simulink model "sin_input1"  (Simulink 7.9)
settings: Solver=ode45  StartTime=0.0  StopTime=50000  RelTol=1e-3
undefined symbols (not defined in the file or in its folder): sfp
libraries: xbsIndex_r4 x2567, xps_library x13, casper_library_misc x4
(part 2 of 28)

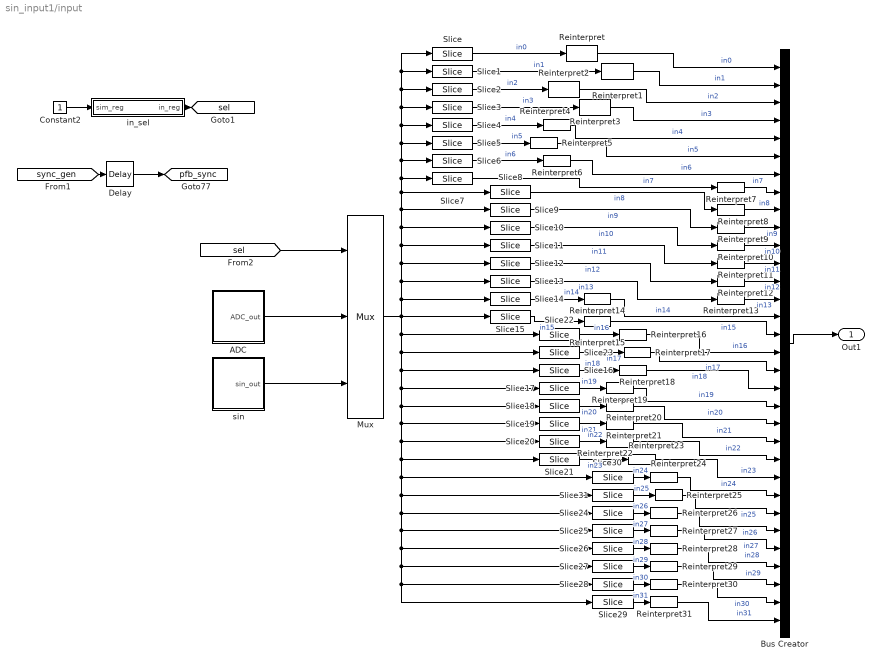
== system sin_input1/input ==
  ADC : Subsystem
  Bus Creator : BusCreator  Inputs=32
  Constant2 : Constant
  Delay : xbsIndex_r4/Delay  rst=off  infoeditControl=Selection of Reset will increase slice count due to use of real FFs and instead of SRLs  en=off  latency=3  dbl_ovrd=off  reg_retiming=off  block_type=delay
  Mux : xbsIndex_r4/Mux  inputs=2  en=off  latency=0  precision=Full  arith_type=Unsigned  n_bits=16  bin_pt=14  quantization=Truncate  overflow=Wrap  dbl_ovrd=off  block_type=mux
  Reinterpret{,1..31} (32 alike) : xbsIndex_r4/Reinterpret  force_arith_type=on  arith_type="Signed  (2's comp)"  force_bin_pt=on  bin_pt=7  block_type=reinterpret
  Slice : xbsIndex_r4/Slice  nbits=8  boolean_output=off  mode=Lower Bit Location + Width  bit1=0  base1=MSB of Input  bit0=248  base0=LSB of Input  dbl_ovrd=off  block_type=slice
  Slice1 : xbsIndex_r4/Slice  nbits=8  boolean_output=off  mode=Lower Bit Location + Width  bit1=0  base1=MSB of Input  bit0=240  base0=LSB of Input  dbl_ovrd=off  block_type=slice
  Slice10 : xbsIndex_r4/Slice  nbits=8  boolean_output=off  mode=Lower Bit Location + Width  bit1=0  base1=MSB of Input  bit0=168  base0=LSB of Input  dbl_ovrd=off  block_type=slice
  Slice11 : xbsIndex_r4/Slice  nbits=8  boolean_output=off  mode=Lower Bit Location + Width  bit1=0  base1=MSB of Input  bit0=160  base0=LSB of Input  dbl_ovrd=off  block_type=slice
  Slice12 : xbsIndex_r4/Slice  nbits=8  boolean_output=off  mode=Lower Bit Location + Width  bit1=0  base1=MSB of Input  bit0=152  base0=LSB of Input  dbl_ovrd=off  block_type=slice
  Slice13 : xbsIndex_r4/Slice  nbits=8  boolean_output=off  mode=Lower Bit Location + Width  bit1=0  base1=MSB of Input  bit0=144  base0=LSB of Input  dbl_ovrd=off  block_type=slice
  Slice14 : xbsIndex_r4/Slice  nbits=8  boolean_output=off  mode=Lower Bit Location + Width  bit1=0  base1=MSB of Input  bit0=136  base0=LSB of Input  dbl_ovrd=off  block_type=slice
  Slice15 : xbsIndex_r4/Slice  nbits=8  boolean_output=off  mode=Lower Bit Location + Width  bit1=0  base1=MSB of Input  bit0=128  base0=LSB of Input  dbl_ovrd=off  block_type=slice
  Slice16 : xbsIndex_r4/Slice  nbits=8  boolean_output=off  mode=Lower Bit Location + Width  bit1=0  base1=MSB of Input  bit0=104  base0=LSB of Input  dbl_ovrd=off  block_type=slice
  Slice17 : xbsIndex_r4/Slice  nbits=8  boolean_output=off  mode=Lower Bit Location + Width  bit1=0  base1=MSB of Input  bit0=96  base0=LSB of Input  dbl_ovrd=off  block_type=slice
  Slice18 : xbsIndex_r4/Slice  nbits=8  boolean_output=off  mode=Lower Bit Location + Width  bit1=0  base1=MSB of Input  bit0=88  base0=LSB of Input  dbl_ovrd=off  block_type=slice
  Slice19 : xbsIndex_r4/Slice  nbits=8  boolean_output=off  mode=Lower Bit Location + Width  bit1=0  base1=MSB of Input  bit0=80  base0=LSB of Input  dbl_ovrd=off  block_type=slice
  Slice2 : xbsIndex_r4/Slice  nbits=8  boolean_output=off  mode=Lower Bit Location + Width  bit1=0  base1=MSB of Input  bit0=232  base0=LSB of Input  dbl_ovrd=off  block_type=slice
  Slice20 : xbsIndex_r4/Slice  nbits=8  boolean_output=off  mode=Lower Bit Location + Width  bit1=0  base1=MSB of Input  bit0=72  base0=LSB of Input  dbl_ovrd=off  block_type=slice
  Slice21 : xbsIndex_r4/Slice  nbits=8  boolean_output=off  mode=Lower Bit Location + Width  bit1=0  base1=MSB of Input  bit0=64  base0=LSB of Input  dbl_ovrd=off  block_type=slice
  Slice22 : xbsIndex_r4/Slice  nbits=8  boolean_output=off  mode=Lower Bit Location + Width  bit1=0  base1=MSB of Input  bit0=120  base0=LSB of Input  dbl_ovrd=off  block_type=slice
  Slice23 : xbsIndex_r4/Slice  nbits=8  boolean_output=off  mode=Lower Bit Location + Width  bit1=0  base1=MSB of Input  bit0=112  base0=LSB of Input  dbl_ovrd=off  block_type=slice
  Slice24 : xbsIndex_r4/Slice  nbits=8  boolean_output=off  mode=Lower Bit Location + Width  bit1=0  base1=MSB of Input  bit0=40  base0=LSB of Input  dbl_ovrd=off  block_type=slice
  Slice25 : xbsIndex_r4/Slice  nbits=8  boolean_output=off  mode=Lower Bit Location + Width  bit1=0  base1=MSB of Input  bit0=32  base0=LSB of Input  dbl_ovrd=off  block_type=slice
  Slice26 : xbsIndex_r4/Slice  nbits=8  boolean_output=off  mode=Lower Bit Location + Width  bit1=0  base1=MSB of Input  bit0=24  base0=LSB of Input  dbl_ovrd=off  block_type=slice
  Slice27 : xbsIndex_r4/Slice  nbits=8  boolean_output=off  mode=Lower Bit Location + Width  bit1=0  base1=MSB of Input  bit0=16  base0=LSB of Input  dbl_ovrd=off  block_type=slice
  Slice28 : xbsIndex_r4/Slice  nbits=8  boolean_output=off  mode=Lower Bit Location + Width  bit1=0  base1=MSB of Input  bit0=8  base0=LSB of Input  dbl_ovrd=off  block_type=slice
  Slice29 : xbsIndex_r4/Slice  nbits=8  boolean_output=off  mode=Lower Bit Location + Width  bit1=0  base1=MSB of Input  bit0=0  base0=LSB of Input  dbl_ovrd=off  block_type=slice
  Slice3 : xbsIndex_r4/Slice  nbits=8  boolean_output=off  mode=Lower Bit Location + Width  bit1=0  base1=MSB of Input  bit0=224  base0=LSB of Input  dbl_ovrd=off  block_type=slice
  Slice30 : xbsIndex_r4/Slice  nbits=8  boolean_output=off  mode=Lower Bit Location + Width  bit1=0  base1=MSB of Input  bit0=56  base0=LSB of Input  dbl_ovrd=off  block_type=slice
  Slice31 : xbsIndex_r4/Slice  nbits=8  boolean_output=off  mode=Lower Bit Location + Width  bit1=0  base1=MSB of Input  bit0=48  base0=LSB of Input  dbl_ovrd=off  block_type=slice
  Slice4 : xbsIndex_r4/Slice  nbits=8  boolean_output=off  mode=Lower Bit Location + Width  bit1=0  base1=MSB of Input  bit0=216  base0=LSB of Input  dbl_ovrd=off  block_type=slice
  Slice5 : xbsIndex_r4/Slice  nbits=8  boolean_output=off  mode=Lower Bit Location + Width  bit1=0  base1=MSB of Input  bit0=208  base0=LSB of Input  dbl_ovrd=off  block_type=slice
  Slice6 : xbsIndex_r4/Slice  nbits=8  boolean_output=off  mode=Lower Bit Location + Width  bit1=0  base1=MSB of Input  bit0=200  base0=LSB of Input  dbl_ovrd=off  block_type=slice
  Slice7 : xbsIndex_r4/Slice  nbits=8  boolean_output=off  mode=Lower Bit Location + Width  bit1=0  base1=MSB of Input  bit0=192  base0=LSB of Input  dbl_ovrd=off  block_type=slice
  Slice8 : xbsIndex_r4/Slice  nbits=8  boolean_output=off  mode=Lower Bit Location + Width  bit1=0  base1=MSB of Input  bit0=184  base0=LSB of Input  dbl_ovrd=off  block_type=slice
  Slice9 : xbsIndex_r4/Slice  nbits=8  boolean_output=off  mode=Lower Bit Location + Width  bit1=0  base1=MSB of Input  bit0=176  base0=LSB of Input  dbl_ovrd=off  block_type=slice
  in_sel : Subsystem  Tag=xps:sw_reg  AncestorBlock=xps_library/software register  mask{numios=1, io_dir=From Processor, io_delay=0, sample_period=1, name1=reg, bitwidth1=1, arith_type1=Boolean, bin_pt1=0}
      mask prompts: numios "Num I/Os"; io_dir "I/O direction"; io_delay "I/O delay"; sample_period "Sample period"; name1 "Name"; bitwidth1 "Bitwidth"; arith_type1 "Type"; bin_pt1 "Binary pt"
      mask init:
      | swreg_init(gcb);
      mask StyleString: edit,popup(To Processor|From Processor),edit,edit,edit,edit,popup(Unsigned|Signed  (2's comp)|Boolean),edit
      mask CallbackString:
      | t = timer('StartDelay', 0.1); t.TimerFcn = {@swreg_cb, gcb}; start(t);|a = get_param(gcb, 'MaskEnables');
      | if strcmp(get_param(gcb, 'io_dir'), 'To Processor'),
      | a(4) = {'off'};
      | else
      | a(4) = {'on'};
      | end
      | set_param(gcb, 'MaskEnables', a);
      | |||||a = get_param(gcb, 'MaskEnables');
      | if strcmp(get_param(gcb, 'arith_type1'), 'Boolean'),
      | set_param(gcb, 'bitwidth1', '1');
      | set_param(gcb, 'bin_pt1', '0');
      | a(6) = {'off'};
      | a(8) = {'off'};
      | else
      | a(6) = {'on'};
      | a(8) = {'on'};
      | end
      | set_param(gcb, 'MaskEnables', a);
      | |
      mask TabNameString: Setup,Setup,Setup,Setup,IO1,IO1,IO1,IO1
  sin : Subsystem
  Out1 : Outport
  tag scope: pfb_sync=global, sel=global
  wires:
  ADC/1(ADC_out) -> Mux/2
  sin/1(sin_out) -> Mux/3
  Mux/1 -> Slice15/1, Slice/1, Slice1/1, Slice2/1, Slice3/1, Slice4/1, Slice5/1, Slice6/1, Slice7/1, Slice8/1, Slice9/1, Slice10/1, Slice11/1, Slice12/1, Slice13/1, Slice14/1, Slice22/1, Slice23/1, Slice16/1, Slice17/1, Slice18/1, Slice19/1, Slice20/1, Slice21/1, Slice30/1, Slice31/1, Slice24/1, Slice25/1, Slice26/1, Slice27/1, Slice29/1, Slice28/1
  Slice/1 -> Reinterpret/1  "in0"
  Slice1/1 -> Reinterpret1/1  "in1"
  Slice2/1 -> Reinterpret2/1  "in2"
  Slice3/1 -> Reinterpret3/1  "in3"
  Slice4/1 -> Reinterpret4/1  "in4"
  Slice5/1 -> Reinterpret5/1  "in5"
  Slice6/1 -> Reinterpret6/1  "in6"
  Slice7/1 -> Reinterpret7/1  "in7"
  Slice8/1 -> Reinterpret8/1  "in8"
  Slice9/1 -> Reinterpret9/1  "in9"
  Slice10/1 -> Reinterpret10/1  "in10"
  Slice11/1 -> Reinterpret11/1  "in11"
  Slice12/1 -> Reinterpret12/1  "in12"
  Slice13/1 -> Reinterpret13/1  "in13"
  Slice14/1 -> Reinterpret14/1  "in14"
  Slice15/1 -> Reinterpret15/1  "in15"
  Slice22/1 -> Reinterpret16/1  "in16"
  Slice17/1 -> Reinterpret19/1  "in19"
  Slice18/1 -> Reinterpret20/1  "in20"
  Slice19/1 -> Reinterpret21/1  "in21"
  Slice20/1 -> Reinterpret22/1  "in22"
  Slice21/1 -> Reinterpret23/1  "in23"
  Slice30/1 -> Reinterpret24/1  "in24"
  Slice31/1 -> Reinterpret25/1  "in25"
  Slice24/1 -> Reinterpret26/1  "in26"
  Slice25/1 -> Reinterpret27/1  "in27"
  Slice26/1 -> Reinterpret28/1  "in28"
  Slice27/1 -> Reinterpret29/1  "in29"
  Slice28/1 -> Reinterpret30/1  "in30"
  Slice29/1 -> Reinterpret31/1  "in31"
  Bus Creator/1 -> Out1/1
  Slice23/1 -> Reinterpret17/1  "in17"
  Slice16/1 -> Reinterpret18/1  "in18"
  Reinterpret/1 -> Bus Creator/1  "in0"
  Reinterpret1/1 -> Bus Creator/2  "in1"
  Reinterpret2/1 -> Bus Creator/3  "in2"
  Reinterpret3/1 -> Bus Creator/4  "in3"
  Reinterpret4/1 -> Bus Creator/5  "in4"
  Reinterpret5/1 -> Bus Creator/6  "in5"
  Reinterpret6/1 -> Bus Creator/7  "in6"
  Reinterpret7/1 -> Bus Creator/8  "in7"
  Reinterpret8/1 -> Bus Creator/9  "in8"
  Reinterpret9/1 -> Bus Creator/10  "in9"
  Reinterpret10/1 -> Bus Creator/11  "in10"
  Reinterpret11/1 -> Bus Creator/12  "in11"
  Reinterpret12/1 -> Bus Creator/13  "in12"
  Reinterpret13/1 -> Bus Creator/14  "in13"
  Reinterpret14/1 -> Bus Creator/15  "in14"
  Reinterpret15/1 -> Bus Creator/16  "in15"
  Reinterpret16/1 -> Bus Creator/17  "in16"
  Reinterpret17/1 -> Bus Creator/18  "in17"
  Reinterpret18/1 -> Bus Creator/19  "in18"
  Reinterpret19/1 -> Bus Creator/20  "in19"
  Reinterpret20/1 -> Bus Creator/21  "in20"
  Reinterpret21/1 -> Bus Creator/22  "in21"
  Reinterpret22/1 -> Bus Creator/23  "in22"
  Reinterpret23/1 -> Bus Creator/24  "in23"
  Reinterpret24/1 -> Bus Creator/25  "in24"
  Reinterpret25/1 -> Bus Creator/26  "in25"
  Reinterpret26/1 -> Bus Creator/27  "in26"
  Reinterpret27/1 -> Bus Creator/28  "in27"
  Reinterpret28/1 -> Bus Creator/29  "in28"
  Reinterpret29/1 -> Bus Creator/30  "in29"
  Reinterpret30/1 -> Bus Creator/31  "in30"
  Reinterpret31/1 -> Bus Creator/32  "in31"
  Delay/1 -> [pfb_sync]
  [sync_gen] -> Delay/1
  Constant2/1 -> in_sel/1(sim_reg)
  in_sel/1(in_reg) -> [sel]
  [sel] -> Mux/1

== system sin_input1/monitor ==
  note: GPIO Select (sync_out)
      | 0: zero
      | 1: sync_gpio_in
      | 2: sync_in
      | 3: adc_clip
      | 4: eq_clip
  note: LED Select
      | 0: Clip (LED1=adc_clip; LED0=eq_clip
      | 1: Sync (LED1=sync_gpio_in; LED0=sync_in)
  note: sel[4] = ledsel
      | sel[2:0] = gpiosel
  BitBasher : xbsIndex_r4/BitBasher  bitexpr=|  display_expr=off  sr_1=1  arith_type1=Unsigned  bin_pt1=0  sr_2=2  arith_type2=Unsigned  bin_pt2=0  sr_3=3  arith_type3=Unsigned  bin_pt3=0  sr_4=4  arith_type4=Unsigned  bin_pt4=0  dbl_ovrd=off  block_type=bitbasher
      | <!DOCTYPE HTML PUBLIC "-//W3C//DTD HTML 4.0//EN" "http://www.w3.org/TR/REC-html40/strict.dtd">
      | <html><head><meta name="qrichtext" content="1" /><style type="text/css">
      | p, li { white-space: pre-wrap; }
      | </style></head><body style=" font-family:'Sans'; font-size:10pt; font-weight:400; font-style:normal;">
      | <p style=" margin-top:12px; margin-bottom:12px; margin-left:0px; margin-right:0px; -qt-block-indent:0; text-indent:0px;"><span style=" font-family:'MS Shell Dlg'; font-size:8pt;">gpiosel={sel[2:0]}<br />ledsel={sel[4]}</span></p></body></html>
  Constant1 : Constant  Value=0
  gpio : Subsystem
  led : Subsystem
  sel : Subsystem  Tag=xps:sw_reg  AncestorBlock=xps_library/software register  mask{numios=1, io_dir=From Processor, io_delay=0, sample_period=1, name1=reg, bitwidth1=32, arith_type1=Unsigned, bin_pt1=0}
      mask prompts: numios "Num I/Os"; io_dir "I/O direction"; io_delay "I/O delay"; sample_period "Sample period"; name1 "Name"; bitwidth1 "Bitwidth"; arith_type1 "Type"; bin_pt1 "Binary pt"
      mask init:
      | swreg_init(gcb);
      mask StyleString: edit,popup(To Processor|From Processor),edit,edit,edit,edit,popup(Unsigned|Signed  (2's comp)|Boolean),edit
      mask CallbackString:
      | t = timer('StartDelay', 0.1); t.TimerFcn = {@swreg_cb, gcb}; start(t);|a = get_param(gcb, 'MaskEnables');
      | if strcmp(get_param(gcb, 'io_dir'), 'To Processor'),
      | a(4) = {'off'};
      | else
      | a(4) = {'on'};
      | end
      | set_param(gcb, 'MaskEnables', a);
      | |||||a = get_param(gcb, 'MaskEnables');
      | if strcmp(get_param(gcb, 'arith_type1'), 'Boolean'),
      | set_param(gcb, 'bitwidth1', '1');
      | set_param(gcb, 'bin_pt1', '0');
      | a(6) = {'off'};
      | a(8) = {'off'};
      | else
      | a(6) = {'on'};
      | a(8) = {'on'};
      | end
      | set_param(gcb, 'MaskEnables', a);
      | |
      mask TabNameString: Setup,Setup,Setup,Setup,IO1,IO1,IO1,IO1
  wires:
  Constant1/1 -> sel/1(sim_reg)
  sel/1(in_reg) -> BitBasher/1
  BitBasher/1 -> [gpiosel]
  BitBasher/2 -> [ledsel]
  [gpiosel] -> gpio/1(sel)
  [ledsel] -> led/1(sel)

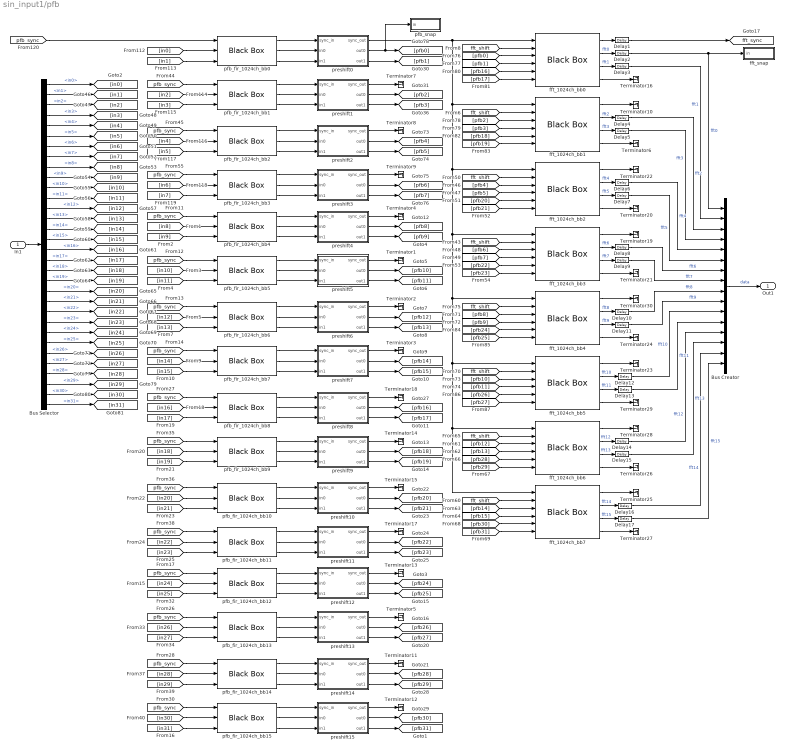
== system sin_input1/pfb ==
  In1 : Inport
  Bus Creator : BusCreator  Inputs=16
  Bus Selector : BusSelector  OutputSignals=in0,in1,in2,in3,in4,in5,in6,in7,in8,in9,in10,in11,in12,in13,in14,in15,in16,in17,in18,in19,in20,in21,in22,in23,in24,in25,in26,in27,in28,in29,in30,in31
  Delay{1..17} (17 alike) : xbsIndex_r4/Delay  rst=off  infoeditControl=Selection of Reset will increase slice count due to use of real FFs and instead of SRLs  en=off  latency=1  dbl_ovrd=off  reg_retiming=off  block_type=delay
  Terminator{1..30} (30 alike) : Terminator
  fft_1024ch_bb{0..7} (8 alike) : xbsIndex_r4/Black Box  init_code=fft_1024ch_core_config  sim_method=Inactive  verbose=off  block_type=blackbox2
  fft_snap : Subsystem
  pfb_fir_1024ch_bb{0..15} (16 alike) : xbsIndex_r4/Black Box  init_code=pfb_fir_1024ch_core_config  sim_method=Inactive  verbose=off  block_type=blackbox2
  pfb_snap : Subsystem
  preshift0 : Subsystem
  preshift1 : Subsystem
  preshift10 : Subsystem
  preshift11 : Subsystem
  preshift12 : Subsystem
  preshift13 : Subsystem
  preshift14 : Subsystem
  preshift15 : Subsystem
  preshift2 : Subsystem
  preshift3 : Subsystem
  preshift4 : Subsystem
  preshift5 : Subsystem
  preshift6 : Subsystem
  preshift7 : Subsystem
  preshift8 : Subsystem
  preshift9 : Subsystem
  Out1 : Outport
  tag scope: fft_sync=global
  wires:
  [in0] -> pfb_fir_1024ch_bb0/2
  [in1] -> pfb_fir_1024ch_bb0/3
  [in2] -> pfb_fir_1024ch_bb1/2
  [in3] -> pfb_fir_1024ch_bb1/3
  [in4] -> pfb_fir_1024ch_bb2/2
  [in5] -> pfb_fir_1024ch_bb2/3
  [in6] -> pfb_fir_1024ch_bb3/2
  [in7] -> pfb_fir_1024ch_bb3/3
  pfb_fir_1024ch_bb1/2 -> preshift1/2(in0)
  pfb_fir_1024ch_bb1/3 -> preshift1/3(in1)
  pfb_fir_1024ch_bb2/2 -> preshift2/2(in0)
  pfb_fir_1024ch_bb2/3 -> preshift2/3(in1)
  pfb_fir_1024ch_bb3/2 -> preshift3/2(in0)
  pfb_fir_1024ch_bb3/3 -> preshift3/3(in1)
  [fft_shift] -> fft_1024ch_bb1/2
  fft_1024ch_bb1/4 -> Terminator6/1
  fft_1024ch_bb0/4 -> Terminator16/1
  Delay1/1 -> [fft_sync]
  fft_1024ch_bb1/1 -> Terminator10/1
  [fft_shift] -> fft_1024ch_bb0/2
  [pfb_sync] -> pfb_fir_1024ch_bb0/1
  [pfb_sync] -> pfb_fir_1024ch_bb1/1
  [pfb_sync] -> pfb_fir_1024ch_bb2/1
  [pfb_sync] -> pfb_fir_1024ch_bb3/1
  pfb_fir_1024ch_bb1/1 -> preshift1/1(sync_in)
  pfb_fir_1024ch_bb2/1 -> preshift2/1(sync_in)
  pfb_fir_1024ch_bb3/1 -> preshift3/1(sync_in)
  pfb_fir_1024ch_bb0/1 -> preshift0/1(sync_in)
  pfb_fir_1024ch_bb0/2 -> preshift0/2(in0)
  pfb_fir_1024ch_bb0/3 -> preshift0/3(in1)
  preshift0/1(sync_out) -> fft_1024ch_bb6/1, fft_1024ch_bb7/1, fft_1024ch_bb5/1, fft_1024ch_bb4/1, fft_1024ch_bb3/1, fft_1024ch_bb2/1, fft_1024ch_bb1/1, fft_1024ch_bb0/1
  preshift0/2(out0) -> [pfb0], pfb_snap/1(in)
  preshift0/3(out1) -> [pfb1]
  preshift1/1(sync_out) -> Terminator7/1
  preshift2/1(sync_out) -> Terminator8/1
  preshift3/1(sync_out) -> Terminator9/1
  preshift1/2(out0) -> [pfb2]
  preshift1/3(out1) -> [pfb3]
  preshift2/2(out0) -> [pfb4]
  preshift2/3(out1) -> [pfb5]
  preshift3/2(out0) -> [pfb6]
  preshift3/3(out1) -> [pfb7]
  [pfb0] -> fft_1024ch_bb0/3
  [pfb1] -> fft_1024ch_bb0/4
  [pfb16] -> fft_1024ch_bb0/5
  [pfb17] -> fft_1024ch_bb0/6
  [pfb2] -> fft_1024ch_bb1/3
  [pfb3] -> fft_1024ch_bb1/4
  [pfb18] -> fft_1024ch_bb1/5
  [pfb19] -> fft_1024ch_bb1/6
  [in8] -> pfb_fir_1024ch_bb4/2
  [in9] -> pfb_fir_1024ch_bb4/3
  [in10] -> pfb_fir_1024ch_bb5/2
  [in11] -> pfb_fir_1024ch_bb5/3
  [in12] -> pfb_fir_1024ch_bb6/2
  [in13] -> pfb_fir_1024ch_bb6/3
  [in14] -> pfb_fir_1024ch_bb7/2
  [in15] -> pfb_fir_1024ch_bb7/3
  pfb_fir_1024ch_bb5/2 -> preshift5/2(in0)
  pfb_fir_1024ch_bb5/3 -> preshift5/3(in1)
  pfb_fir_1024ch_bb6/2 -> preshift6/2(in0)
  pfb_fir_1024ch_bb6/3 -> preshift6/3(in1)
  pfb_fir_1024ch_bb7/2 -> preshift7/2(in0)
  pfb_fir_1024ch_bb7/3 -> preshift7/3(in1)
  [pfb_sync] -> pfb_fir_1024ch_bb4/1
  [pfb_sync] -> pfb_fir_1024ch_bb5/1
  [pfb_sync] -> pfb_fir_1024ch_bb6/1
  [pfb_sync] -> pfb_fir_1024ch_bb7/1
  pfb_fir_1024ch_bb5/1 -> preshift5/1(sync_in)
  pfb_fir_1024ch_bb6/1 -> preshift6/1(sync_in)
  pfb_fir_1024ch_bb7/1 -> preshift7/1(sync_in)
  pfb_fir_1024ch_bb4/1 -> preshift4/1(sync_in)
  pfb_fir_1024ch_bb4/2 -> preshift4/2(in0)
  pfb_fir_1024ch_bb4/3 -> preshift4/3(in1)
  preshift4/1(sync_out) -> Terminator4/1
  preshift4/2(out0) -> [pfb8]
  preshift4/3(out1) -> [pfb9]
  preshift5/1(sync_out) -> Terminator1/1
  preshift6/1(sync_out) -> Terminator2/1
  preshift7/1(sync_out) -> Terminator3/1
  preshift5/2(out0) -> [pfb10]
  preshift5/3(out1) -> [pfb11]
  preshift6/2(out0) -> [pfb12]
  preshift6/3(out1) -> [pfb13]
  preshift7/2(out0) -> [pfb14]
  preshift7/3(out1) -> [pfb15]
  [in16] -> pfb_fir_1024ch_bb8/2
  [in17] -> pfb_fir_1024ch_bb8/3
  [in18] -> pfb_fir_1024ch_bb9/2
  [in19] -> pfb_fir_1024ch_bb9/3
  [in20] -> pfb_fir_1024ch_bb10/2
  [in21] -> pfb_fir_1024ch_bb10/3
  [in22] -> pfb_fir_1024ch_bb11/2
  [in23] -> pfb_fir_1024ch_bb11/3
  pfb_fir_1024ch_bb9/2 -> preshift9/2(in0)
  pfb_fir_1024ch_bb9/3 -> preshift9/3(in1)
  pfb_fir_1024ch_bb10/2 -> preshift10/2(in0)
  pfb_fir_1024ch_bb10/3 -> preshift10/3(in1)
  pfb_fir_1024ch_bb11/2 -> preshift11/2(in0)
  pfb_fir_1024ch_bb11/3 -> preshift11/3(in1)
  [pfb_sync] -> pfb_fir_1024ch_bb8/1
  [pfb_sync] -> pfb_fir_1024ch_bb9/1
  [pfb_sync] -> pfb_fir_1024ch_bb10/1
  [pfb_sync] -> pfb_fir_1024ch_bb11/1
  pfb_fir_1024ch_bb9/1 -> preshift9/1(sync_in)
  pfb_fir_1024ch_bb10/1 -> preshift10/1(sync_in)
  pfb_fir_1024ch_bb11/1 -> preshift11/1(sync_in)
  pfb_fir_1024ch_bb8/1 -> preshift8/1(sync_in)
  pfb_fir_1024ch_bb8/2 -> preshift8/2(in0)
  pfb_fir_1024ch_bb8/3 -> preshift8/3(in1)
  preshift8/1(sync_out) -> Terminator18/1
  preshift8/2(out0) -> [pfb16]
  preshift8/3(out1) -> [pfb17]
  preshift9/1(sync_out) -> Terminator14/1
  preshift10/1(sync_out) -> Terminator15/1
  preshift11/1(sync_out) -> Terminator17/1
  preshift9/2(out0) -> [pfb18]
  preshift9/3(out1) -> [pfb19]
  preshift10/2(out0) -> [pfb20]
  preshift10/3(out1) -> [pfb21]
  preshift11/2(out0) -> [pfb22]
  preshift11/3(out1) -> [pfb23]
  [in24] -> pfb_fir_1024ch_bb12/2
  [in25] -> pfb_fir_1024ch_bb12/3
  [in26] -> pfb_fir_1024ch_bb13/2
  [in27] -> pfb_fir_1024ch_bb13/3
  [in28] -> pfb_fir_1024ch_bb14/2
  [in29] -> pfb_fir_1024ch_bb14/3
  [in30] -> pfb_fir_1024ch_bb15/2
  [in31] -> pfb_fir_1024ch_bb15/3
  pfb_fir_1024ch_bb13/2 -> preshift13/2(in0)
  pfb_fir_1024ch_bb13/3 -> preshift13/3(in1)
  pfb_fir_1024ch_bb14/2 -> preshift14/2(in0)
  pfb_fir_1024ch_bb14/3 -> preshift14/3(in1)
  pfb_fir_1024ch_bb15/2 -> preshift15/2(in0)
  pfb_fir_1024ch_bb15/3 -> preshift15/3(in1)
  [pfb_sync] -> pfb_fir_1024ch_bb12/1
  [pfb_sync] -> pfb_fir_1024ch_bb13/1
  [pfb_sync] -> pfb_fir_1024ch_bb14/1
  [pfb_sync] -> pfb_fir_1024ch_bb15/1
  pfb_fir_1024ch_bb13/1 -> preshift13/1(sync_in)
  pfb_fir_1024ch_bb14/1 -> preshift14/1(sync_in)
  pfb_fir_1024ch_bb15/1 -> preshift15/1(sync_in)
  pfb_fir_1024ch_bb12/1 -> preshift12/1(sync_in)
  pfb_fir_1024ch_bb12/2 -> preshift12/2(in0)
  pfb_fir_1024ch_bb12/3 -> preshift12/3(in1)
  preshift12/1(sync_out) -> Terminator13/1
  preshift12/2(out0) -> [pfb24]
  preshift12/3(out1) -> [pfb25]
  preshift13/1(sync_out) -> Terminator5/1
  preshift14/1(sync_out) -> Terminator11/1
  preshift15/1(sync_out) -> Terminator12/1
  preshift13/2(out0) -> [pfb26]
  preshift13/3(out1) -> [pfb27]
  preshift14/2(out0) -> [pfb28]
  preshift14/3(out1) -> [pfb29]
  preshift15/2(out0) -> [pfb30]
  preshift15/3(out1) -> [pfb31]
  [fft_shift] -> fft_1024ch_bb3/2
  fft_1024ch_bb3/4 -> Terminator21/1
  fft_1024ch_bb2/4 -> Terminator20/1
  fft_1024ch_bb2/1 -> Terminator22/1
  fft_1024ch_bb3/1 -> Terminator19/1
  [fft_shift] -> fft_1024ch_bb2/2
  [pfb4] -> fft_1024ch_bb2/3
  [pfb5] -> fft_1024ch_bb2/4
  [pfb20] -> fft_1024ch_bb2/5
  [pfb21] -> fft_1024ch_bb2/6
  [pfb6] -> fft_1024ch_bb3/3
  [pfb7] -> fft_1024ch_bb3/4
  [pfb22] -> fft_1024ch_bb3/5
  [pfb23] -> fft_1024ch_bb3/6
  [fft_shift] -> fft_1024ch_bb5/2
  fft_1024ch_bb5/4 -> Terminator29/1
  fft_1024ch_bb4/4 -> Terminator24/1
  fft_1024ch_bb4/1 -> Terminator30/1
  fft_1024ch_bb5/1 -> Terminator23/1
  [fft_shift] -> fft_1024ch_bb4/2
  [pfb8] -> fft_1024ch_bb4/3
  [pfb9] -> fft_1024ch_bb4/4
  [pfb24] -> fft_1024ch_bb4/5
  [pfb25] -> fft_1024ch_bb4/6
  [pfb10] -> fft_1024ch_bb5/3
  [pfb11] -> fft_1024ch_bb5/4
  [pfb26] -> fft_1024ch_bb5/5
  [pfb27] -> fft_1024ch_bb5/6
  [fft_shift] -> fft_1024ch_bb7/2
  fft_1024ch_bb7/4 -> Terminator27/1
  fft_1024ch_bb6/4 -> Terminator26/1
  fft_1024ch_bb6/1 -> Terminator28/1
  fft_1024ch_bb7/1 -> Terminator25/1
  [fft_shift] -> fft_1024ch_bb6/2
  [pfb12] -> fft_1024ch_bb6/3
  [pfb13] -> fft_1024ch_bb6/4
  [pfb28] -> fft_1024ch_bb6/5
  [pfb29] -> fft_1024ch_bb6/6
  [pfb14] -> fft_1024ch_bb7/3
  [pfb15] -> fft_1024ch_bb7/4
  [pfb30] -> fft_1024ch_bb7/5
  [pfb31] -> fft_1024ch_bb7/6
  In1/1 -> Bus Selector/1
  Bus Selector/1 -> [in0]  "<in0>"
  Bus Selector/2 -> [in1]  "<in1>"
  Bus Selector/3 -> [in2]  "<in2>"
  Bus Selector/4 -> [in3]  "<in3>"
  Delay2/1 -> Bus Creator/1, fft_snap/1(in)  "fft0"
  Delay3/1 -> Bus Creator/2  "fft1"
  Delay4/1 -> Bus Creator/3  "fft2"
  Delay5/1 -> Bus Creator/4  "fft3"
  Delay6/1 -> Bus Creator/5  "fft4"
  Delay7/1 -> Bus Creator/6  "fft5"
  Delay8/1 -> Bus Creator/7  "fft6"
  Delay9/1 -> Bus Creator/8  "fft7"
  Delay10/1 -> Bus Creator/9  "fft8"
  Delay11/1 -> Bus Creator/10  "fft9"
  Delay12/1 -> Bus Creator/11  "fft10"
  Delay13/1 -> Bus Creator/12  "fft11"
  Delay14/1 -> Bus Creator/13  "fft12"
  Delay15/1 -> Bus Creator/14  "fft13"
  Delay16/1 -> Bus Creator/15  "fft14"
  Delay17/1 -> Bus Creator/16  "fft15"
  Bus Creator/1 -> Out1/1  "data"
  fft_1024ch_bb7/2 -> Delay16/1  "fft14"
  fft_1024ch_bb7/3 -> Delay17/1  "fft15"
  fft_1024ch_bb6/2 -> Delay14/1  "fft12"
  fft_1024ch_bb6/3 -> Delay15/1  "fft13"
  fft_1024ch_bb5/2 -> Delay12/1  "fft10"
  fft_1024ch_bb5/3 -> Delay13/1  "fft11"
  fft_1024ch_bb0/2 -> Delay2/1  "fft0"
  fft_1024ch_bb0/1 -> Delay1/1
  fft_1024ch_bb0/3 -> Delay3/1  "fft1"
  fft_1024ch_bb1/2 -> Delay4/1  "fft2"
  fft_1024ch_bb1/3 -> Delay5/1  "fft3"
  fft_1024ch_bb2/2 -> Delay6/1  "fft4"
  fft_1024ch_bb2/3 -> Delay7/1  "fft5"
  fft_1024ch_bb3/2 -> Delay8/1  "fft6"
  fft_1024ch_bb3/3 -> Delay9/1  "fft7"
  fft_1024ch_bb4/2 -> Delay10/1  "fft8"
  fft_1024ch_bb4/3 -> Delay11/1  "fft9"
  Bus Selector/5 -> [in4]  "<in4>"
  Bus Selector/6 -> [in5]  "<in5>"
  Bus Selector/7 -> [in6]  "<in6>"
  Bus Selector/8 -> [in7]  "<in7>"
  Bus Selector/9 -> [in8]  "<in8>"
  Bus Selector/10 -> [in9]  "<in9>"
  Bus Selector/11 -> [in10]  "<in10>"
  Bus Selector/12 -> [in11]  "<in11>"
  Bus Selector/13 -> [in12]  "<in12>"
  Bus Selector/14 -> [in13]  "<in13>"
  Bus Selector/15 -> [in14]  "<in14>"
  Bus Selector/16 -> [in15]  "<in15>"
  Bus Selector/17 -> [in16]  "<in16>"
  Bus Selector/18 -> [in17]  "<in17>"
  Bus Selector/19 -> [in18]  "<in18>"
  Bus Selector/20 -> [in19]  "<in19>"
  Bus Selector/21 -> [in20]  "<in20>"
  Bus Selector/22 -> [in21]  "<in21>"
  Bus Selector/23 -> [in22]  "<in22>"
  Bus Selector/24 -> [in23]  "<in23>"
  Bus Selector/25 -> [in24]  "<in24>"
  Bus Selector/26 -> [in25]  "<in25>"
  Bus Selector/27 -> [in26]  "<in26>"
  Bus Selector/28 -> [in27]  "<in27>"
  Bus Selector/29 -> [in28]  "<in28>"
  Bus Selector/30 -> [in29]  "<in29>"
  Bus Selector/31 -> [in30]  "<in30>"
  Bus Selector/32 -> [in31]  "<in31>"

== system sin_input1/rcs ==
  note: 0 -> revision info
      | 1 -> timestamp
  note: Last modification
      | in seconds since
      | 01-01-1970
  note: 0 -> git
      | 1 -> svn
  note: 0 -> cleanly in revision control
  note: git -> first 28 bits of commit hash
      | svn -> latest revision number
  note: 0 -> revision info
      | 1 -> timestamp
  note: Compile time
      | in seconds since
      | 01-01-1970
  note: 0 -> git
      | 1 -> svn
  note: 0 -> cleanly in revision control
  note: git -> first 28 bits of commit hash
      | svn -> latest revision number
  Concat : xbsIndex_r4/Concat  num_inputs=2  dbl_ovrd=off  block_type=concat
  Concat1 : xbsIndex_r4/Concat  num_inputs=2  dbl_ovrd=off  block_type=concat
  Mux : xbsIndex_r4/Mux  inputs=2  en=off  latency=0  precision=Full  arith_type=Unsigned  n_bits=16  bin_pt=14  quantization=Truncate  overflow=Wrap  dbl_ovrd=off  block_type=mux
      settings: block_version=11.4
  Mux1 : xbsIndex_r4/Mux  inputs=2  en=off  latency=0  precision=Full  arith_type=Unsigned  n_bits=16  bin_pt=14  quantization=Truncate  overflow=Wrap  dbl_ovrd=off  block_type=mux
      settings: block_version=11.4
  Terminator : Terminator
  Terminator1 : Terminator
  Terminator2 : Terminator
  app : Subsystem  Tag=xps:sw_reg  mask{io_dir=To Processor, arith_type=Unsigned, bitwidth=32, bin_pt=0, sample_period=1, latency=0}
      mask prompts: io_dir "I/O direction"; arith_type "Data Type"; bitwidth "Data bitwidth"; bin_pt "Data binary point"; sample_period "Sample period"
      mask init:
      | gw_name = clear_name(gcb);
      | config.source = str2func('software_register_init');
      | config.toplevel = gcb;
      | xBlock( config, {io_dir, arith_type, bitwidth, bin_pt, ... 
      |     sample_period, gw_name, latency});
      mask StyleString: popup(From Processor|To Processor),popup(Unsigned|Signed  (2's comp)),edit,edit,edit,edit
  app_dirty : xbsIndex_r4/Constant  const=1  gui_display_data_type=Fixed-point  arith_type=Unsigned  n_bits=1  bin_pt=0  preci_type=Single  exp_width=8  frac_width=24  explicit_period=on  period=1  equ=P=C  opselect=C  inp2=PCIN>>17  opr=+  inp1=P  carry=CIN  dbl_ovrd=off  block_type=constant
      settings: block_version=11.4
  app_info : xbsIndex_r4/Concat  num_inputs=4  dbl_ovrd=off  block_type=concat
  app_info1 : xbsIndex_r4/Concat  num_inputs=4  dbl_ovrd=off  block_type=concat
  app_rcs_type : xbsIndex_r4/Constant  const=0  gui_display_data_type=Fixed-point  arith_type=Unsigned  n_bits=1  bin_pt=0  preci_type=Single  exp_width=8  frac_width=24  explicit_period=on  period=1  equ=P=C  opselect=C  inp2=PCIN>>17  opr=+  inp1=P  carry=CIN  dbl_ovrd=off  block_type=constant
      settings: block_version=11.4
  app_reserved : xbsIndex_r4/Constant  const=0  gui_display_data_type=Fixed-point  arith_type=Unsigned  n_bits=1  bin_pt=0  preci_type=Single  exp_width=8  frac_width=24  explicit_period=on  period=1  equ=P=C  opselect=C  inp2=PCIN>>17  opr=+  inp1=P  carry=CIN  dbl_ovrd=off  block_type=constant
      settings: block_version=11.4
  app_revision : xbsIndex_r4/Constant  const=0  gui_display_data_type=Fixed-point  arith_type=Unsigned  n_bits=28  bin_pt=0  preci_type=Single  exp_width=8  frac_width=24  explicit_period=on  period=1  equ=P=C  opselect=C  inp2=PCIN>>17  opr=+  inp1=P  carry=CIN  dbl_ovrd=off  block_type=constant
      settings: block_version=11.4
  app_timestamp : xbsIndex_r4/Constant  const=1445516336  gui_display_data_type=Fixed-point  arith_type=Unsigned  n_bits=31  bin_pt=0  preci_type=Single  exp_width=8  frac_width=24  explicit_period=on  period=1  equ=P=C  opselect=C  inp2=PCIN>>17  opr=+  inp1=P  carry=CIN  dbl_ovrd=off  block_type=constant
      settings: block_version=11.4
  app_type : xbsIndex_r4/Constant  const=1  gui_display_data_type=Fixed-point  arith_type=Unsigned  n_bits=1  bin_pt=0  preci_type=Single  exp_width=8  frac_width=24  explicit_period=on  period=1  equ=P=C  opselect=C  inp2=PCIN>>17  opr=+  inp1=P  carry=CIN  dbl_ovrd=off  block_type=constant
      settings: block_version=11.4
  lib : Subsystem  Tag=xps:sw_reg  mask{io_dir=To Processor, arith_type=Unsigned, bitwidth=32, bin_pt=0, sample_period=1, latency=0}
      mask prompts: io_dir "I/O direction"; arith_type "Data Type"; bitwidth "Data bitwidth"; bin_pt "Data binary point"; sample_period "Sample period"
      mask init:
      | gw_name = clear_name(gcb);
      | config.source = str2func('software_register_init');
      | config.toplevel = gcb;
      | xBlock( config, {io_dir, arith_type, bitwidth, bin_pt, ... 
      |     sample_period, gw_name, latency});
      mask StyleString: popup(From Processor|To Processor),popup(Unsigned|Signed  (2's comp)),edit,edit,edit,edit
  lib_dirty : xbsIndex_r4/Constant  const=1  gui_display_data_type=Fixed-point  arith_type=Unsigned  n_bits=1  bin_pt=0  preci_type=Single  exp_width=8  frac_width=24  explicit_period=on  period=1  equ=P=C  opselect=C  inp2=PCIN>>17  opr=+  inp1=P  carry=CIN  dbl_ovrd=off  block_type=constant
      settings: block_version=11.4
  lib_rcs_type : xbsIndex_r4/Constant  const=0  gui_display_data_type=Fixed-point  arith_type=Unsigned  n_bits=1  bin_pt=0  preci_type=Single  exp_width=8  frac_width=24  explicit_period=on  period=1  equ=P=C  opselect=C  inp2=PCIN>>17  opr=+  inp1=P  carry=CIN  dbl_ovrd=off  block_type=constant
      settings: block_version=11.4
  lib_reserved : xbsIndex_r4/Constant  const=0  gui_display_data_type=Fixed-point  arith_type=Unsigned  n_bits=1  bin_pt=0  preci_type=Single  exp_width=8  frac_width=24  explicit_period=on  period=1  equ=P=C  opselect=C  inp2=PCIN>>17  opr=+  inp1=P  carry=CIN  dbl_ovrd=off  block_type=constant
      settings: block_version=11.4
  lib_revision : xbsIndex_r4/Constant  const=hex2dec('2f40dad')  gui_display_data_type=Fixed-point  arith_type=Unsigned  n_bits=28  bin_pt=0  preci_type=Single  exp_width=8  frac_width=24  explicit_period=on  period=1  equ=P=C  opselect=C  inp2=PCIN>>17  opr=+  inp1=P  carry=CIN  dbl_ovrd=off  block_type=constant
      settings: block_version=11.4
  lib_timestamp : xbsIndex_r4/Constant  const=1445545304  gui_display_data_type=Fixed-point  arith_type=Unsigned  n_bits=31  bin_pt=0  preci_type=Single  exp_width=8  frac_width=24  explicit_period=on  period=1  equ=P=C  opselect=C  inp2=PCIN>>17  opr=+  inp1=P  carry=CIN  dbl_ovrd=off  block_type=constant
      settings: block_version=11.4
  lib_type : xbsIndex_r4/Constant  const=0  gui_display_data_type=Fixed-point  arith_type=Unsigned  n_bits=1  bin_pt=0  preci_type=Single  exp_width=8  frac_width=24  explicit_period=on  period=1  equ=P=C  opselect=C  inp2=PCIN>>17  opr=+  inp1=P  carry=CIN  dbl_ovrd=off  block_type=constant
      settings: block_version=11.4
  user : Subsystem  Tag=xps:sw_reg  mask{io_dir=To Processor, arith_type=Unsigned, bitwidth=32, bin_pt=0, sample_period=1, latency=0}
      mask prompts: io_dir "I/O direction"; arith_type "Data Type"; bitwidth "Data bitwidth"; bin_pt "Data binary point"; sample_period "Sample period"
      mask init:
      | gw_name = clear_name(gcb);
      | config.source = str2func('software_register_init');
      | config.toplevel = gcb;
      | xBlock( config, {io_dir, arith_type, bitwidth, bin_pt, ... 
      |     sample_period, gw_name, latency});
      mask StyleString: popup(From Processor|To Processor),popup(Unsigned|Signed  (2's comp)),edit,edit,edit,edit
  user_val : xbsIndex_r4/Constant  const=user_val  gui_display_data_type=Fixed-point  arith_type=Unsigned  n_bits=32  bin_pt=0  preci_type=Single  exp_width=8  frac_width=24  explicit_period=off  period=1  equ=P=C  opselect=C  inp2=PCIN>>17  opr=+  inp1=P  carry=CIN  dbl_ovrd=off  block_type=constant
      settings: block_version=11.4
  wires:
  app/1(sim_reg_out) -> Terminator/1
  user/1(sim_reg_out) -> Terminator2/1
  user_val/1 -> user/1(reg_out)
  app_rcs_type/1 -> app_info/1
  app_reserved/1 -> app_info/2
  app_dirty/1 -> app_info/3
  app_revision/1 -> app_info/4
  app_type/1 -> Concat/1, Mux/1
  app_info/1 -> Mux/2
  app_timestamp/1 -> Mux/3
  Mux/1 -> Concat/2
  Concat/1 -> app/1(reg_out)
  lib/1(sim_reg_out) -> Terminator1/1
  lib_rcs_type/1 -> app_info1/1
  lib_reserved/1 -> app_info1/2
  lib_dirty/1 -> app_info1/3
  lib_revision/1 -> app_info1/4
  lib_type/1 -> Concat1/1, Mux1/1
  app_info1/1 -> Mux1/2
  lib_timestamp/1 -> Mux1/3
  Mux1/1 -> Concat1/2
  Concat1/1 -> lib/1(reg_out)

== system sin_input1/seed ==
  0 : Subsystem  Tag=xps:sw_reg  AncestorBlock=xps_library/software register  mask{numios=1, io_dir=From Processor, io_delay=0, sample_period=1, name1=reg, bitwidth1=32, arith_type1=Unsigned, bin_pt1=0}
      mask prompts: numios "Num I/Os"; io_dir "I/O direction"; io_delay "I/O delay"; sample_period "Sample period"; name1 "Name"; bitwidth1 "Bitwidth"; arith_type1 "Type"; bin_pt1 "Binary pt"
      mask init:
      | swreg_init(gcb);
      mask StyleString: edit,popup(To Processor|From Processor),edit,edit,edit,edit,popup(Unsigned|Signed  (2's comp)|Boolean),edit
      mask CallbackString:
      | t = timer('StartDelay', 0.1); t.TimerFcn = {@swreg_cb, gcb}; start(t);|a = get_param(gcb, 'MaskEnables');
      | if strcmp(get_param(gcb, 'io_dir'), 'To Processor'),
      | a(4) = {'off'};
      | else
      | a(4) = {'on'};
      | end
      | set_param(gcb, 'MaskEnables', a);
      | |||||a = get_param(gcb, 'MaskEnables');
      | if strcmp(get_param(gcb, 'arith_type1'), 'Boolean'),
      | set_param(gcb, 'bitwidth1', '1');
      | set_param(gcb, 'bin_pt1', '0');
      | a(6) = {'off'};
      | a(8) = {'off'};
      | else
      | a(6) = {'on'};
      | a(8) = {'on'};
      | end
      | set_param(gcb, 'MaskEnables', a);
      | |
      mask TabNameString: Setup,Setup,Setup,Setup,IO1,IO1,IO1,IO1
  1 : Subsystem  Tag=xps:sw_reg  AncestorBlock=xps_library/software register  mask{numios=1, io_dir=From Processor, io_delay=0, sample_period=1, name1=reg, bitwidth1=32, arith_type1=Unsigned, bin_pt1=0}
      mask prompts: numios "Num I/Os"; io_dir "I/O direction"; io_delay "I/O delay"; sample_period "Sample period"; name1 "Name"; bitwidth1 "Bitwidth"; arith_type1 "Type"; bin_pt1 "Binary pt"
      mask init:
      | swreg_init(gcb);
      mask StyleString: edit,popup(To Processor|From Processor),edit,edit,edit,edit,popup(Unsigned|Signed  (2's comp)|Boolean),edit
      mask CallbackString:
      | t = timer('StartDelay', 0.1); t.TimerFcn = {@swreg_cb, gcb}; start(t);|a = get_param(gcb, 'MaskEnables');
      | if strcmp(get_param(gcb, 'io_dir'), 'To Processor'),
      | a(4) = {'off'};
      | else
      | a(4) = {'on'};
      | end
      | set_param(gcb, 'MaskEnables', a);
      | |||||a = get_param(gcb, 'MaskEnables');
      | if strcmp(get_param(gcb, 'arith_type1'), 'Boolean'),
      | set_param(gcb, 'bitwidth1', '1');
      | set_param(gcb, 'bin_pt1', '0');
      | a(6) = {'off'};
      | a(8) = {'off'};
      | else
      | a(6) = {'on'};
      | a(8) = {'on'};
      | end
      | set_param(gcb, 'MaskEnables', a);
      | |
      mask TabNameString: Setup,Setup,Setup,Setup,IO1,IO1,IO1,IO1
  2 : Subsystem  Tag=xps:sw_reg  AncestorBlock=xps_library/software register  mask{numios=1, io_dir=From Processor, io_delay=0, sample_period=1, name1=reg, bitwidth1=32, arith_type1=Unsigned, bin_pt1=0}
      mask prompts: numios "Num I/Os"; io_dir "I/O direction"; io_delay "I/O delay"; sample_period "Sample period"; name1 "Name"; bitwidth1 "Bitwidth"; arith_type1 "Type"; bin_pt1 "Binary pt"
      mask init:
      | swreg_init(gcb);
      mask StyleString: edit,popup(To Processor|From Processor),edit,edit,edit,edit,popup(Unsigned|Signed  (2's comp)|Boolean),edit
      mask CallbackString:
      | t = timer('StartDelay', 0.1); t.TimerFcn = {@swreg_cb, gcb}; start(t);|a = get_param(gcb, 'MaskEnables');
      | if strcmp(get_param(gcb, 'io_dir'), 'To Processor'),
      | a(4) = {'off'};
      | else
      | a(4) = {'on'};
      | end
      | set_param(gcb, 'MaskEnables', a);
      | |||||a = get_param(gcb, 'MaskEnables');
      | if strcmp(get_param(gcb, 'arith_type1'), 'Boolean'),
      | set_param(gcb, 'bitwidth1', '1');
      | set_param(gcb, 'bin_pt1', '0');
      | a(6) = {'off'};
      | a(8) = {'off'};
      | else
      | a(6) = {'on'};
      | a(8) = {'on'};
      | end
      | set_param(gcb, 'MaskEnables', a);
      | |
      mask TabNameString: Setup,Setup,Setup,Setup,IO1,IO1,IO1,IO1
  3 : Subsystem  Tag=xps:sw_reg  AncestorBlock=xps_library/software register  mask{numios=1, io_dir=From Processor, io_delay=0, sample_period=1, name1=reg, bitwidth1=32, arith_type1=Unsigned, bin_pt1=0}
      mask prompts: numios "Num I/Os"; io_dir "I/O direction"; io_delay "I/O delay"; sample_period "Sample period"; name1 "Name"; bitwidth1 "Bitwidth"; arith_type1 "Type"; bin_pt1 "Binary pt"
      mask init:
      | swreg_init(gcb);
      mask StyleString: edit,popup(To Processor|From Processor),edit,edit,edit,edit,popup(Unsigned|Signed  (2's comp)|Boolean),edit
      mask CallbackString:
      | t = timer('StartDelay', 0.1); t.TimerFcn = {@swreg_cb, gcb}; start(t);|a = get_param(gcb, 'MaskEnables');
      | if strcmp(get_param(gcb, 'io_dir'), 'To Processor'),
      | a(4) = {'off'};
      | else
      | a(4) = {'on'};
      | end
      | set_param(gcb, 'MaskEnables', a);
      | |||||a = get_param(gcb, 'MaskEnables');
      | if strcmp(get_param(gcb, 'arith_type1'), 'Boolean'),
      | set_param(gcb, 'bitwidth1', '1');
      | set_param(gcb, 'bin_pt1', '0');
      | a(6) = {'off'};
      | a(8) = {'off'};
      | else
      | a(6) = {'on'};
      | a(8) = {'on'};
      | end
      | set_param(gcb, 'MaskEnables', a);
      | |
      mask TabNameString: Setup,Setup,Setup,Setup,IO1,IO1,IO1,IO1
  BitBasher : xbsIndex_r4/BitBasher  bitexpr=|  display_expr=off  sr_1=1  arith_type1=Unsigned  bin_pt1=0  sr_2=2  arith_type2=Unsigned  bin_pt2=0  sr_3=3  arith_type3=Unsigned  bin_pt3=0  sr_4=4  arith_type4=Unsigned  bin_pt4=0  dbl_ovrd=off  block_type=bitbasher
      | <!DOCTYPE HTML PUBLIC "-//W3C//DTD HTML 4.0//EN" "http://www.w3.org/TR/REC-html40/strict.dtd">
      | <html><head><meta name="qrichtext" content="1" /><style type="text/css">
      | p, li { white-space: pre-wrap; }
      | </style></head><body style=" font-family:'Sans'; font-size:10pt; font-weight:400; font-style:normal;">
      | <p style=" margin-top:12px; margin-bottom:12px; margin-left:0px; margin-right:0px; -qt-block-indent:0; text-indent:0px;"><span style=" font-family:'MS Shell Dlg'; font-size:8pt;">s0={8'h5c, s32[7:0], s32[0:7], 8'ha3}<br />s1={8'h5c, s32[15:8], s32[8:15], 8'ha3}<br />s2={8'h5c, s32[23:16], s32[16:23], 8'ha3}<br />s3={8'h5c, s32[31:24], s32[24:31], 8'ha3}</span></p></body></html>
  BitBasher1 : xbsIndex_r4/BitBasher  bitexpr=|  display_expr=off  sr_1=1  arith_type1=Unsigned  bin_pt1=0  sr_2=2  arith_type2=Unsigned  bin_pt2=0  sr_3=3  arith_type3=Unsigned  bin_pt3=0  sr_4=4  arith_type4=Unsigned  bin_pt4=0  dbl_ovrd=off  block_type=bitbasher
      | <!DOCTYPE HTML PUBLIC "-//W3C//DTD HTML 4.0//EN" "http://www.w3.org/TR/REC-html40/strict.dtd">
      | <html><head><meta name="qrichtext" content="1" /><style type="text/css">
      | p, li { white-space: pre-wrap; }
      | </style></head><body style=" font-family:'Sans'; font-size:10pt; font-weight:400; font-style:normal;">
      | <p style=" margin-top:12px; margin-bottom:12px; margin-left:0px; margin-right:0px; -qt-block-indent:0; text-indent:0px;"><span style=" font-family:'MS Shell Dlg'; font-size:8pt;">s0={8'h5c, s32[7:0], s32[0:7], 8'ha3}<br />s1={8'h5c, s32[15:8], s32[8:15], 8'ha3}<br />s2={8'h5c, s32[23:16], s32[16:23], 8'ha3}<br />s3={8'h5c, s32[31:24], s32[24:31], 8'ha3}</span></p></body></html>
  BitBasher2 : xbsIndex_r4/BitBasher  bitexpr=|  display_expr=off  sr_1=1  arith_type1=Unsigned  bin_pt1=0  sr_2=2  arith_type2=Unsigned  bin_pt2=0  sr_3=3  arith_type3=Unsigned  bin_pt3=0  sr_4=4  arith_type4=Unsigned  bin_pt4=0  dbl_ovrd=off  block_type=bitbasher
      | <!DOCTYPE HTML PUBLIC "-//W3C//DTD HTML 4.0//EN" "http://www.w3.org/TR/REC-html40/strict.dtd">
      | <html><head><meta name="qrichtext" content="1" /><style type="text/css">
      | p, li { white-space: pre-wrap; }
      | </style></head><body style=" font-family:'Sans'; font-size:10pt; font-weight:400; font-style:normal;">
      | <p style=" margin-top:12px; margin-bottom:12px; margin-left:0px; margin-right:0px; -qt-block-indent:0; text-indent:0px;"><span style=" font-family:'MS Shell Dlg'; font-size:8pt;">s0={8'h5c, s32[7:0], s32[0:7], 8'ha3}<br />s1={8'h5c, s32[15:8], s32[8:15], 8'ha3}<br />s2={8'h5c, s32[23:16], s32[16:23], 8'ha3}<br />s3={8'h5c, s32[31:24], s32[24:31], 8'ha3}</span></p></body></html>
  BitBasher3 : xbsIndex_r4/BitBasher  bitexpr=|  display_expr=off  sr_1=1  arith_type1=Unsigned  bin_pt1=0  sr_2=2  arith_type2=Unsigned  bin_pt2=0  sr_3=3  arith_type3=Unsigned  bin_pt3=0  sr_4=4  arith_type4=Unsigned  bin_pt4=0  dbl_ovrd=off  block_type=bitbasher
      | <!DOCTYPE HTML PUBLIC "-//W3C//DTD HTML 4.0//EN" "http://www.w3.org/TR/REC-html40/strict.dtd">
      | <html><head><meta name="qrichtext" content="1" /><style type="text/css">
      | p, li { white-space: pre-wrap; }
      | </style></head><body style=" font-family:'Sans'; font-size:10pt; font-weight:400; font-style:normal;">
      | <p style=" margin-top:12px; margin-bottom:12px; margin-left:0px; margin-right:0px; -qt-block-indent:0; text-indent:0px;"><span style=" font-family:'MS Shell Dlg'; font-size:8pt;">s0={8'h5c, s32[7:0], s32[0:7], 8'ha3}<br />s1={8'h5c, s32[15:8], s32[8:15], 8'ha3}<br />s2={8'h5c, s32[23:16], s32[16:23], 8'ha3}<br />s3={8'h5c, s32[31:24], s32[24:31], 8'ha3}</span></p></body></html>
  Constant{,1..3} (4 alike) : Constant  Value=0
  armed_trigger : casper_library_misc/armed_trigger
  tag scope: seed0=global, seed10=global, seed11=global, seed12=global, seed13=global, seed14=global, seed15=global, seed1=global, seed2=global, seed3=global, seed4=global, seed5=global, seed6=global, seed7=global, seed8=global, seed9=global, seed_ld=global
  wires:
  Constant3/1 -> 3/1(sim_reg)
  Constant2/1 -> 2/1(sim_reg)
  Constant1/1 -> 1/1(sim_reg)
  1/1(in_reg) -> BitBasher1/1
  Constant/1 -> 0/1(sim_reg)
  0/1(in_reg) -> BitBasher/1
  BitBasher/1 -> [seed0]
  BitBasher/2 -> [seed1]
  BitBasher/3 -> [seed2]
  BitBasher/4 -> [seed3]
  BitBasher1/1 -> [seed4]
  BitBasher1/2 -> [seed5]
  BitBasher1/3 -> [seed6]
  BitBasher1/4 -> [seed7]
  BitBasher2/1 -> [seed8]
  BitBasher2/2 -> [seed9]
  BitBasher2/3 -> [seed10]
  BitBasher2/4 -> [seed11]
  BitBasher3/1 -> [seed12]
  BitBasher3/2 -> [seed13]
  BitBasher3/3 -> [seed14]
  BitBasher3/4 -> [seed15]
  3/1(in_reg) -> BitBasher3/1
  2/1(in_reg) -> BitBasher2/1
  [arm_noise] -> armed_trigger/1
  [sync_in] -> armed_trigger/2
  armed_trigger/1 -> [seed_ld]

== system sin_input1/snap ==
  0 : Subsystem  Tag=casper:snapshot  AncestorBlock=casper_library_scopes/snapshot  mask(snapshot){storage=bram, dram_dimm=1, dram_clock=200, nsamples=12, data_width=32, offset=off, circap=off, value=off, use_dsp48=off}
      mask prompts: storage "Storage medium"; dram_dimm "DRAM dimm"; dram_clock "DRAM clock rate"; nsamples "Number of Samples (2^?)"; data_width "Data width"; offset "Start delay support"; circap "Circular capture support"; value "Extra value capture support"; use_dsp48 "Use DSP48s to implement counters"
      mask help:
      | Standard snap block, but with the following optional extras;
      |  1) Optionally delay the fabric trigger by a user-specified number of valids.
      |  2) Capture continuously after a trigger until a stop pulse is received. Data is captured into BRAM continuously, with old data being overwritten until a stop command is received. Then stop capturing when memory is filled. This enables you to get some data before and some data after the stop pulse. Use the 'tr_en_cnt' register to determine where you are in your vector. 'Addr' register's MSb now indicates 'busy capturing'.
      |  3) Optionally capture a value on an input port to a register at the same time as the first data sample.
      |  4) Implement counters using DSP48Es to save resources.
      mask init:
      | snapshot_init(gcb, ...
      |     'storage', storage, ...
      |     'dram_dimm', dram_dimm, ...
      |     'dram_clock', dram_clock, ...
      |     'nsamples', nsamples, ...
      |     'data_width', data_width, ...
      |     'offset', offset, ...
      |     'circap', circap, ...
      |     'value', value, ...
      |     'use_dsp48', use_dsp48);
      mask StyleString: popup(bram|dram),popup(1|2|3|4),popup(200|250|266|300|333),edit,popup(8|16|32|64|128),checkbox,checkbox,checkbox,checkbox
      mask CallbackString: snapshot_callback();||||||||
  0_sel : Subsystem  Tag=xps:sw_reg  AncestorBlock=xps_library/software register  mask{numios=1, io_dir=From Processor, io_delay=0, sample_period=1, name1=reg, bitwidth1=32, arith_type1=Unsigned, bin_pt1=0}
      mask prompts: numios "Num I/Os"; io_dir "I/O direction"; io_delay "I/O delay"; sample_period "Sample period"; name1 "Name"; bitwidth1 "Bitwidth"; arith_type1 "Type"; bin_pt1 "Binary pt"
      mask init:
      | swreg_init(gcb);
      mask StyleString: edit,popup(To Processor|From Processor),edit,edit,edit,edit,popup(Unsigned|Signed  (2's comp)|Boolean),edit
      mask CallbackString:
      | t = timer('StartDelay', 0.1); t.TimerFcn = {@swreg_cb, gcb}; start(t);|a = get_param(gcb, 'MaskEnables');
      | if strcmp(get_param(gcb, 'io_dir'), 'To Processor'),
      | a(4) = {'off'};
      | else
      | a(4) = {'on'};
      | end
      | set_param(gcb, 'MaskEnables', a);
      | |||||a = get_param(gcb, 'MaskEnables');
      | if strcmp(get_param(gcb, 'arith_type1'), 'Boolean'),
      | set_param(gcb, 'bitwidth1', '1');
      | set_param(gcb, 'bin_pt1', '0');
      | a(6) = {'off'};
      | a(8) = {'off'};
      | else
      | a(6) = {'on'};
      | a(8) = {'on'};
      | end
      | set_param(gcb, 'MaskEnables', a);
      | |
      mask TabNameString: Setup,Setup,Setup,Setup,IO1,IO1,IO1,IO1
  BitBasher : xbsIndex_r4/BitBasher  bitexpr=|  display_expr=off  sr_1=1  arith_type1=Unsigned  bin_pt1=0  sr_2=2  arith_type2=Unsigned  bin_pt2=0  sr_3=3  arith_type3=Unsigned  bin_pt3=0  sr_4=4  arith_type4=Unsigned  bin_pt4=0  dbl_ovrd=off  block_type=bitbasher
      | <!DOCTYPE HTML PUBLIC "-//W3C//DTD HTML 4.0//EN" "http://www.w3.org/TR/REC-html40/strict.dtd">
      | <html><head><meta name="qrichtext" content="1" /><style type="text/css">
      | p, li { white-space: pre-wrap; }
      | </style></head><body style=" font-family:'Sans'; font-size:10pt; font-weight:400; font-style:normal;">
      | <p style=" margin-top:12px; margin-bottom:12px; margin-left:0px; margin-right:0px; -qt-block-indent:0; text-indent:0px;"><span style=" font-family:'MS Shell Dlg'; font-size:8pt;">sel={reg[1:0]}</span></p></body></html>
  Constant : Constant  Value=0
  Constant1 : xbsIndex_r4/Constant  const=1  gui_display_data_type=Fixed-point  arith_type=Signed (2's comp)  n_bits=16  bin_pt=14  preci_type=Single  exp_width=8  frac_width=24  explicit_period=off  period=1  equ=P=C  opselect=C  inp2=PCIN>>17  opr=+  inp1=P  carry=CIN  dbl_ovrd=off  block_type=constant
  Mux : xbsIndex_r4/Mux  inputs=4  en=off  latency=1  precision=Full  arith_type=Unsigned  n_bits=16  bin_pt=14  quantization=Truncate  overflow=Wrap  dbl_ovrd=off  block_type=mux
  zero : xbsIndex_r4/Constant  const=0  gui_display_data_type=Fixed-point  arith_type=Boolean  n_bits=16  bin_pt=14  preci_type=Single  exp_width=8  frac_width=24  explicit_period=on  period=1  equ=P=C  opselect=C  inp2=PCIN>>17  opr=+  inp1=P  carry=CIN  dbl_ovrd=off  block_type=constant
      settings: block_version=11.4
  wires:
  Constant/1 -> 0_sel/1(sim_reg)
  0_sel/1(in_reg) -> BitBasher/1
  Constant1/1 -> Mux/2
  [pfb_snap] -> Mux/3
  [fft_snap] -> Mux/4
  [eq_snap] -> Mux/5
  BitBasher/1 -> Mux/1
  Mux/1 -> 0/1(din)
  zero/1 -> 0/2(we), 0/3(trig)
